simulink model "gate_V29_2016a"  (R2016a)
settings: Solver=FixedStepDiscrete  StartTime=0.0  StopTime=100  FixedStep=0.001  RelTol=1e-3
values from neighbouring files (symbols this model uses):
  CLOSE_CHAR = CHAR_STAR    [GateParameter.m]
  CLOSE_SIDE_VALUE = single(4*ONE_SEC)    [GateParameter.m]
  CLOSED = uint8(12)    [GateParameter.m]
  CLOSING = uint8(13)    [GateParameter.m]
  NO_PASS_ASSIG = uint8(6)    [GateParameter.m]
  OPEN = uint8(14)    [GateParameter.m]
  OPEN_CHAR = CHAR_HASH    [GateParameter.m]
  OPEN_SIDE_VALUE = uint16(4*ONE_SEC)    [GateParameter.m]
  OPENING = uint8(11)    [GateParameter.m]
  OPENING_ACTIVE = uint8(1)    [GateParameter.m]
  PASS_ASSIGNED = uint8(7)    [GateParameter.m]
  PASS_INCOMPLETE = uint8(8)    [GateParameter.m]
  PASS_TIMEOUT = uint16(10*ONE_SEC)    [GateParameter.m]
  PASSWORD_VERIFIED = uint8(5)    [GateParameter.m]
  TIMEOUT = uint8(9)    [GateParameter.m]
  WRONG_PASSWORD = uint8(2)    [GateParameter.m]
undefined symbols (not defined in the file or in its folder): OPEN_TIMEOUT, sigbuilder_block
libraries: simulink x9, arduinolib x5
(part 1 of 3)
settings Model: ExtModeTrigDuration=2  Creator=omaro  LastModifiedBy=oelkawok
settings DataIOCC: SaveFormat=StructureWithTime
settings OptimizationCC: DefaultParameterBehavior=Inlined  StateBitsets=on  DataBitsets=on  LifeSpan=1  MaxStackSize=64
settings DebuggingCC: ConsistencyChecking=error  ArrayBoundsChecking=error  SignalInfNanChecking=error  SignalRangeChecking=error  ReadBeforeWriteMsg=EnableAllAsError  WriteAfterWriteMsg=EnableAllAsError  WriteAfterReadMsg=EnableAllAsError  AlgebraicLoopMsg=error  ArtificialAlgebraicLoopMsg=error  BlockPriorityViolationMsg=error  MinStepSizeMsg=error  CheckMatrixSingularityMsg=error  ParameterUnderflowMsg=error  ParameterPrecisionLossMsg=error  UnderSpecifiedDataTypeMsg=warning  UnnecessaryDatatypeConvMsg=warning  VectorMatrixConversionMsg=error  SignalLabelMismatchMsg=error  SFcnCompatibilityMsg=warning  UniqueDataStoreMsg=error  BusObjectLabelMismatch=error  RootOutportRequireBusObject=error  ModelReferenceIOMsg=error  ModelReferenceVersionMismatchMessage=error  ModelReferenceIOMismatchMessage=error  ModelReferenceDataLoggingMessage=error  StrictBusMsg=ErrorOnBusTreatedAsVector  SFUnexpectedBacktrackingDiag=error  SFInvalidInputDataAccessInChartInitDiag=error  SFNoUnconditionalDefaultTransitionDiag=error  SFTransitionOutsideNaturalParentDiag=error  SFUndirectedBroadcastEventsDiag=error  SFTransitionActionBeforeConditionDiag=error  SFOutputUsedAsStateInMooreChartDiag=warning
settings SFSimCC: SimCustomHeaderCode=|
  | //#include "C:\Users\omaro\Desktop\Gate_Model\Arduino\Arduino.h"
  | //#include "Keypad\Keypad.h"
settings RTWCC: SystemTargetFile=ert.tlc  TemplateMakefile=ert_default_tmf  GenerateReport=on  RTWUseSimCustomCode=on  Toolchain=Arduino AVR v1.6.1  BuildConfiguration=Faster Runs  LaunchReport=on
settings RTWCC: CustomHeaderCode=|
  | //#include "C:\Users\omaro\Desktop\Gate_Model\Arduino\Arduino.h"
  | //#include "Keypad\Keypad.h"
settings CodeAppCC: ForceParamTrailComments=on  OperatorAnnotations=on  MATLABSourceComments=on
settings ERTTargetCC: ERTCustomFileBanners=off  SupportContinuousTime=on  SupportNonInlinedSFcns=on  SupportVariableSizeSignals=on  ModelStepFunctionPrototypeControlCompliant=off  RootIOFormat=Structure reference  ERTCustomFileTemplate=example_file_process.tlc

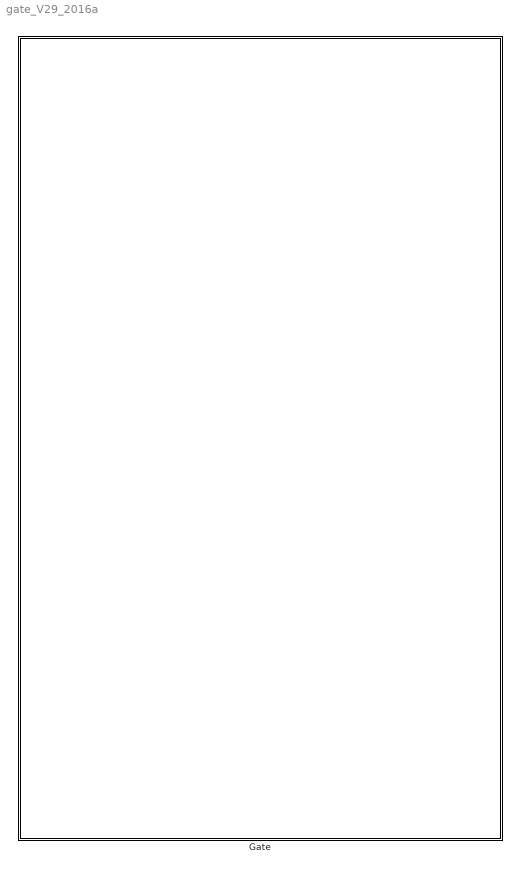
== system gate_V29_2016a ==
  Gate : Subsystem

== system gate_V29_2016a/Gate ==
  GATE : Subsystem  Variant=on  VariantControl=Variant1  OverrideUsingVariant=VariantB
  HardwareInputWrapper : Subsystem  Variant=on  VariantControl=Variant1  OverrideUsingVariant=MIL_VariantB
  outputPinsBlock : Subsystem  Variant=on  VariantControl=Variant1  OverrideUsingVariant=MIL_ALL_Variants
  wires:
  HardwareInputWrapper/9(SimuOutputLimits) -> outputPinsBlock/9(SimuOutputLimits)
  HardwareInputWrapper/2(BtCharReceived) -> GATE/2("BtCharReceived ")  "BtCharReceived_UINT8"
  HardwareInputWrapper/3(PassSwPressedOut) -> GATE/3("PassSwPressedOut ")  "PassSwPressedOut_BOOL"
  HardwareInputWrapper/4(rightCurrentRaw) -> GATE/4(rightCurrentRaw)  "rightSpeedRaw_UINT16"
  HardwareInputWrapper/6(RFPressedOut) -> GATE/6("RFPressedOut ")  "RFPressedOut_UINT8"
  GATE/1(" greenLedPin") -> outputPinsBlock/1(greenLedPin)  "greenLedPin_BOOL"
  GATE/2(" redLedPin") -> outputPinsBlock/2(redLedPin)  "redLedPin_BOOL"
  GATE/3(" yellowLedPin") -> outputPinsBlock/3(yellowLed)  "yellowLedPin_BOOL"
  GATE/4(" LeftMotorPin") -> outputPinsBlock/4(leftMotorPin)  "LeftMotorPin_BOOL"
  GATE/5(" CommonMotorPin") -> outputPinsBlock/5(commonMotorPin)  "ComMotorPin_BOOL"
  GATE/6(" rightMotorPin") -> outputPinsBlock/6(rightMotorPin)  "RightMotorPin_BOOL"
  GATE/7(" bulbPin") -> outputPinsBlock/7(bulbPin)  "bulbPin_BOOL"
  GATE/8(" BT_TxChar") -> outputPinsBlock/8(BT_TxChar)  "BT_TxChar_UINT8"
  HardwareInputWrapper/5(leftCurrentRaw) -> GATE/5(leftCurrentRaw)  "leftSpeedRaw_UINT16"
  HardwareInputWrapper/1(KpCharPressed) -> GATE/1("KpCharPressed ")  "KpCharPressed_UINT8"
  GATE/10(passwordOut) -> [passwordOut]  "passwordRAM_UINT8_6D"
  GATE/9(passAssigned) -> [passAssigned]  "passAssignedRAM_BOOL"
  [passwordOut] -> HardwareInputWrapper/2(passwordIn)
  [passAssigned] -> HardwareInputWrapper/1(passAssigned)
  HardwareInputWrapper/7(passwordOut) -> GATE/7(passwordIn)  "passwordEEPROM_UINT8_6D"
  HardwareInputWrapper/8(passAssignedOut) -> GATE/8(passAssignedIn)  "passAssignedEEPROM_BOOL"

== system gate_V29_2016a/Gate/GATE ==
  "KpCharPressed " : Inport
  "BtCharReceived " : Inport  Port=2
  "PassSwPressedOut " : Inport  Port=3
  rightCurrentRaw : Inport  Port=4
  leftCurrentRaw : Inport  Port=5
  "RFPressedOut " : Inport  Port=6
  passwordIn : Inport  Port=7
  passAssignedIn : Inport  Port=8
  GATE_A : Subsystem  VariantControl=VariantA
  GATE_B : Subsystem  VariantControl=VariantB
  GATE_C : Subsystem  VariantControl=VariantC
  " greenLedPin" : Outport
  " redLedPin" : Outport  Port=2
  " yellowLedPin" : Outport  Port=3
  " LeftMotorPin" : Outport  Port=4
  " CommonMotorPin" : Outport  Port=5
  " rightMotorPin" : Outport  Port=6
  " bulbPin" : Outport  Port=7
  " BT_TxChar" : Outport  Port=8
  passAssigned : Outport  Port=9
  passwordOut : Outport  Port=10

== system gate_V29_2016a/Gate/HardwareInputWrapper ==
  passAssigned : Inport
  passwordIn : Inport  Port=2
  HIL : Subsystem  VariantControl=HardwareInloopInputs
  MIL_Gate_A : Subsystem  VariantControl=MIL_VariantA
  MIL_Gate_B : Subsystem  VariantControl=MIL_VariantB
  MIL_Gate_C : Subsystem  VariantControl=MIL_VariantC
  KpCharPressed : Outport
  BtCharReceived : Outport  Port=2
  PassSwPressedOut : Outport  Port=3
  rightCurrentRaw : Outport  Port=4
  leftCurrentRaw : Outport  Port=5
  RFPressedOut : Outport  Port=6
  passwordOut : Outport  Port=7
  passAssignedOut : Outport  Port=8
  SimuOutputLimits : Outport  Port=9

== system gate_V29_2016a/Gate/outputPinsBlock ==
  greenLedPin : Inport
  redLedPin : Inport  Port=2
  yellowLed : Inport  Port=3
  leftMotorPin : Inport  Port=4
  commonMotorPin : Inport  Port=5
  rightMotorPin : Inport  Port=6
  bulbPin : Inport  Port=7
  BT_TxChar : Inport  Port=8
  SimuOutputLimits : Inport  Port=9
  HIL : Subsystem  VariantControl=HardwareInLoopOutputs
  MIL : Subsystem  Variant=on  VariantControl=MIL_ALL_Variants  OverrideUsingVariant=VisualOutputs

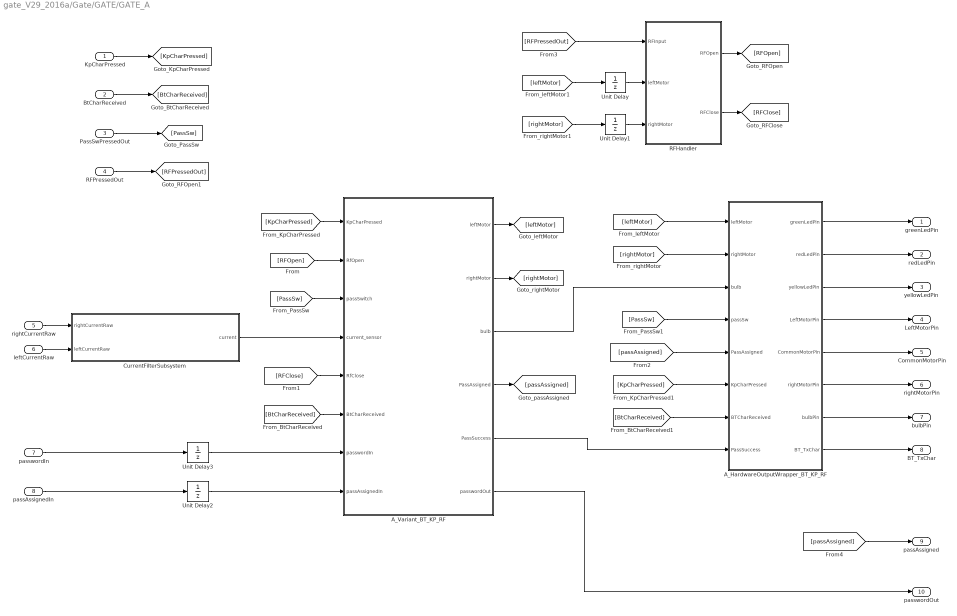
== system gate_V29_2016a/Gate/GATE/GATE_A ==
  KpCharPressed : Inport
  BtCharReceived : Inport  Port=2
  PassSwPressedOut : Inport  Port=3
  RFPressedOut : Inport  Port=4
  rightCurrentRaw : Inport  Port=5
  leftCurrentRaw : Inport  Port=6
  passwordIn : Inport  Port=7
  passAssignedIn : Inport  Port=8
  A_HardwareOutputWrapper_BT_KP_RF : Subsystem  VariantControl=Variant1
  A_Variant_BT_KP_RF : Subsystem  VariantControl=Variant
  CurrentFilterSubsystem : Subsystem
  RFHandler : Subsystem
  Unit Delay{,1..3} (4 alike) : UnitDelay  SampleTime=-1
  greenLedPin : Outport
  redLedPin : Outport  Port=2
  yellowLedPin : Outport  Port=3
  LeftMotorPin : Outport  Port=4
  CommonMotorPin : Outport  Port=5
  rightMotorPin : Outport  Port=6
  bulbPin : Outport  Port=7
  BT_TxChar : Outport  Port=8
  passAssigned : Outport  Port=9
  passwordOut : Outport  Port=10
  wires:
  RFHandler/1(RFOpen) -> [RFOpen]
  RFHandler/2(RFClose) -> [RFClose]
  [RFPressedOut] -> RFHandler/1(RFInput)
  RFPressedOut/1 -> [RFPressedOut]
  PassSwPressedOut/1 -> [PassSw]
  [leftMotor] -> Unit Delay/1
  Unit Delay/1 -> RFHandler/2(leftMotor)
  [rightMotor] -> Unit Delay1/1
  Unit Delay1/1 -> RFHandler/3(rightMotor)
  BtCharReceived/1 -> [BtCharReceived]
  KpCharPressed/1 -> [KpCharPressed]
  [RFOpen] -> A_Variant_BT_KP_RF/2(RfOpen)
  [PassSw] -> A_Variant_BT_KP_RF/3(passSwitch)
  [RFClose] -> A_Variant_BT_KP_RF/5(RfClose)
  [BtCharReceived] -> A_Variant_BT_KP_RF/6(BtCharReceived)
  A_Variant_BT_KP_RF/1(leftMotor) -> [leftMotor]
  A_Variant_BT_KP_RF/2(rightMotor) -> [rightMotor]
  [KpCharPressed] -> A_Variant_BT_KP_RF/1(KpCharPressed)
  [leftMotor] -> A_HardwareOutputWrapper_BT_KP_RF/1(leftMotor)
  [rightMotor] -> A_HardwareOutputWrapper_BT_KP_RF/2(rightMotor)
  A_Variant_BT_KP_RF/3(bulb) -> A_HardwareOutputWrapper_BT_KP_RF/3(bulb)
  [PassSw] -> A_HardwareOutputWrapper_BT_KP_RF/4(passSw)
  [KpCharPressed] -> A_HardwareOutputWrapper_BT_KP_RF/6(KpCharPressed)
  [BtCharReceived] -> A_HardwareOutputWrapper_BT_KP_RF/7(BTCharReceived)
  A_HardwareOutputWrapper_BT_KP_RF/1(greenLedPin) -> greenLedPin/1
  A_HardwareOutputWrapper_BT_KP_RF/2(redLedPin) -> redLedPin/1
  A_HardwareOutputWrapper_BT_KP_RF/3(yellowLedPin) -> yellowLedPin/1
  A_HardwareOutputWrapper_BT_KP_RF/4(LeftMotorPin) -> LeftMotorPin/1
  A_HardwareOutputWrapper_BT_KP_RF/5(CommonMotorPin) -> CommonMotorPin/1
  A_HardwareOutputWrapper_BT_KP_RF/6(rightMotorPin) -> rightMotorPin/1
  A_HardwareOutputWrapper_BT_KP_RF/7(bulbPin) -> bulbPin/1
  A_HardwareOutputWrapper_BT_KP_RF/8(BT_TxChar) -> BT_TxChar/1
  A_Variant_BT_KP_RF/4(PassAssigned) -> [passAssigned]
  [passAssigned] -> A_HardwareOutputWrapper_BT_KP_RF/5(PassAssigned)
  Unit Delay2/1 -> A_Variant_BT_KP_RF/8(passAssignedIn)
  Unit Delay3/1 -> A_Variant_BT_KP_RF/7(passwordIn)
  A_Variant_BT_KP_RF/5(PassSuccess) -> A_HardwareOutputWrapper_BT_KP_RF/8(PassSuccess)
  CurrentFilterSubsystem/1(current) -> A_Variant_BT_KP_RF/4(current_sensor)
  rightCurrentRaw/1 -> CurrentFilterSubsystem/1(rightCurrentRaw)
  leftCurrentRaw/1 -> CurrentFilterSubsystem/2(leftCurrentRaw)
  passAssignedIn/1 -> Unit Delay2/1
  passwordIn/1 -> Unit Delay3/1
  A_Variant_BT_KP_RF/6(passwordOut) -> passwordOut/1
  [passAssigned] -> passAssigned/1

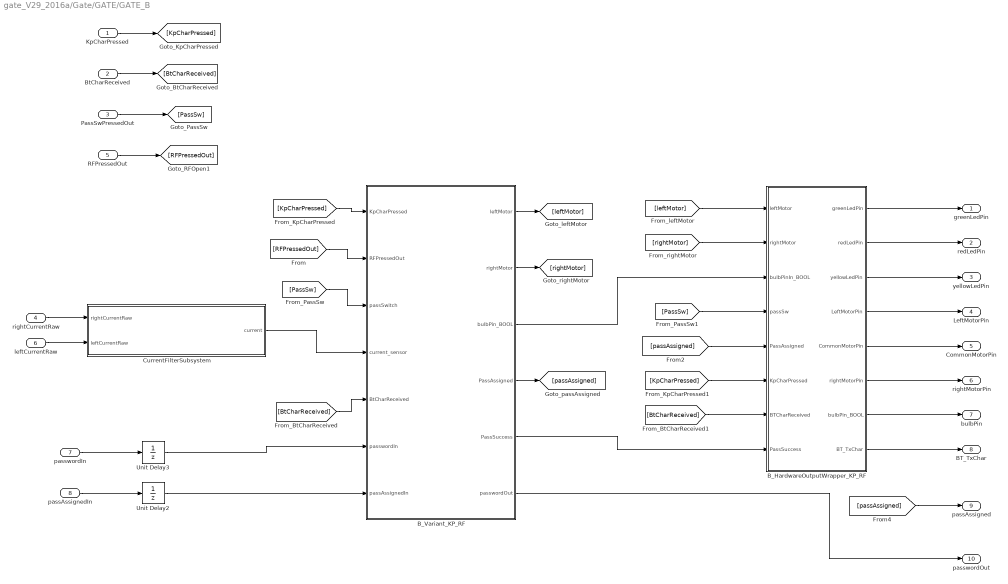
== system gate_V29_2016a/Gate/GATE/GATE_B ==
  KpCharPressed : Inport
  BtCharReceived : Inport  Port=2
  PassSwPressedOut : Inport  Port=3
  rightCurrentRaw : Inport  Port=4
  RFPressedOut : Inport  Port=5
  leftCurrentRaw : Inport  Port=6
  passwordIn : Inport  Port=7
  passAssignedIn : Inport  Port=8
  B_HardwareOutputWrapper_KP_RF : Subsystem  VariantControl=Variant2
  B_Variant_KP_RF : Subsystem  VariantControl=Variant2
  CurrentFilterSubsystem : Subsystem
  Unit Delay2 : UnitDelay  SampleTime=-1
  Unit Delay3 : UnitDelay  SampleTime=-1
  greenLedPin : Outport
  redLedPin : Outport  Port=2
  yellowLedPin : Outport  Port=3
  LeftMotorPin : Outport  Port=4
  CommonMotorPin : Outport  Port=5
  rightMotorPin : Outport  Port=6
  bulbPin : Outport  Port=7
  BT_TxChar : Outport  Port=8
  passAssigned : Outport  Port=9
  passwordOut : Outport  Port=10
  wires:
  CurrentFilterSubsystem/1(current) -> B_Variant_KP_RF/4(current_sensor)
  B_Variant_KP_RF/5(PassSuccess) -> B_HardwareOutputWrapper_KP_RF/8(PassSuccess)
  Unit Delay3/1 -> B_Variant_KP_RF/6(passwordIn)
  Unit Delay2/1 -> B_Variant_KP_RF/7(passAssignedIn)
  B_Variant_KP_RF/6(passwordOut) -> passwordOut/1
  [passAssigned] -> B_HardwareOutputWrapper_KP_RF/5(PassAssigned)
  B_Variant_KP_RF/4(PassAssigned) -> [passAssigned]
  B_HardwareOutputWrapper_KP_RF/8(BT_TxChar) -> BT_TxChar/1
  B_HardwareOutputWrapper_KP_RF/7(bulbPin_BOOL) -> bulbPin/1
  B_HardwareOutputWrapper_KP_RF/6(rightMotorPin) -> rightMotorPin/1
  B_HardwareOutputWrapper_KP_RF/5(CommonMotorPin) -> CommonMotorPin/1
  B_HardwareOutputWrapper_KP_RF/4(LeftMotorPin) -> LeftMotorPin/1
  B_HardwareOutputWrapper_KP_RF/3(yellowLedPin) -> yellowLedPin/1
  B_HardwareOutputWrapper_KP_RF/2(redLedPin) -> redLedPin/1
  B_HardwareOutputWrapper_KP_RF/1(greenLedPin) -> greenLedPin/1
  [BtCharReceived] -> B_HardwareOutputWrapper_KP_RF/7(BTCharReceived)
  [KpCharPressed] -> B_HardwareOutputWrapper_KP_RF/6(KpCharPressed)
  [PassSw] -> B_HardwareOutputWrapper_KP_RF/4(passSw)
  B_Variant_KP_RF/3(bulbPin_BOOL) -> B_HardwareOutputWrapper_KP_RF/3(bulbPinIn_BOOL)
  [rightMotor] -> B_HardwareOutputWrapper_KP_RF/2(rightMotor)
  [leftMotor] -> B_HardwareOutputWrapper_KP_RF/1(leftMotor)
  [KpCharPressed] -> B_Variant_KP_RF/1(KpCharPressed)
  B_Variant_KP_RF/2(rightMotor) -> [rightMotor]
  B_Variant_KP_RF/1(leftMotor) -> [leftMotor]
  [BtCharReceived] -> B_Variant_KP_RF/5(BtCharReceived)
  rightCurrentRaw/1 -> CurrentFilterSubsystem/1(rightCurrentRaw)
  [PassSw] -> B_Variant_KP_RF/3(passSwitch)
  [RFPressedOut] -> B_Variant_KP_RF/2(RFPressedOut)
  KpCharPressed/1 -> [KpCharPressed]
  BtCharReceived/1 -> [BtCharReceived]
  PassSwPressedOut/1 -> [PassSw]
  RFPressedOut/1 -> [RFPressedOut]
  leftCurrentRaw/1 -> CurrentFilterSubsystem/2(leftCurrentRaw)
  [passAssigned] -> passAssigned/1
  passAssignedIn/1 -> Unit Delay2/1
  passwordIn/1 -> Unit Delay3/1

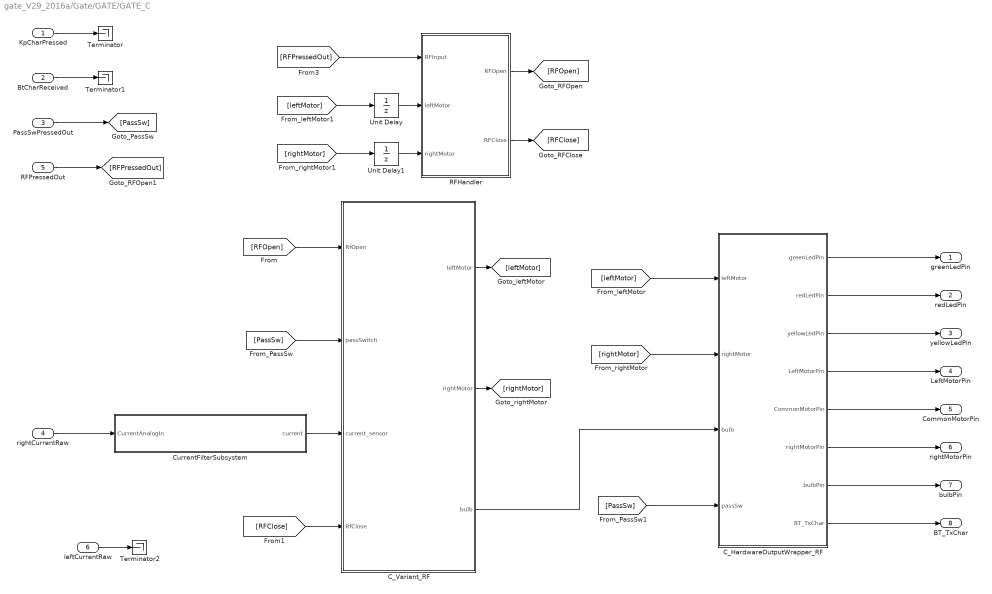
== system gate_V29_2016a/Gate/GATE/GATE_C ==
  KpCharPressed : Inport
  BtCharReceived : Inport  Port=2
  PassSwPressedOut : Inport  Port=3
  rightCurrentRaw : Inport  Port=4
  RFPressedOut : Inport  Port=5
  leftCurrentRaw : Inport  Port=6
  C_HardwareOutputWrapper_RF : Subsystem  VariantControl=Variant3
  C_Variant_RF : Subsystem  VariantControl=Variant3
  CurrentFilterSubsystem : Subsystem
  RFHandler : Subsystem
  Terminator : Terminator
  Terminator1 : Terminator
  Terminator2 : Terminator
  Unit Delay : UnitDelay  SampleTime=-1
  Unit Delay1 : UnitDelay  SampleTime=-1
  greenLedPin : Outport
  redLedPin : Outport  Port=2
  yellowLedPin : Outport  Port=3
  LeftMotorPin : Outport  Port=4
  CommonMotorPin : Outport  Port=5
  rightMotorPin : Outport  Port=6
  bulbPin : Outport  Port=7
  BT_TxChar : Outport  Port=8
  wires:
  RFHandler/1(RFOpen) -> [RFOpen]
  RFHandler/2(RFClose) -> [RFClose]
  [RFPressedOut] -> RFHandler/1(RFInput)
  RFPressedOut/1 -> [RFPressedOut]
  PassSwPressedOut/1 -> [PassSw]
  [leftMotor] -> Unit Delay/1
  Unit Delay/1 -> RFHandler/2(leftMotor)
  [rightMotor] -> Unit Delay1/1
  Unit Delay1/1 -> RFHandler/3(rightMotor)
  BtCharReceived/1 -> Terminator1/1
  KpCharPressed/1 -> Terminator/1
  [RFOpen] -> C_Variant_RF/1(RfOpen)
  [PassSw] -> C_Variant_RF/2(passSwitch)
  rightCurrentRaw/1 -> CurrentFilterSubsystem/1(CurrentAnalogIn)
  [RFClose] -> C_Variant_RF/4(RfClose)
  C_Variant_RF/1(leftMotor) -> [leftMotor]
  C_Variant_RF/2(rightMotor) -> [rightMotor]
  [leftMotor] -> C_HardwareOutputWrapper_RF/1(leftMotor)
  [rightMotor] -> C_HardwareOutputWrapper_RF/2(rightMotor)
  C_Variant_RF/3(bulb) -> C_HardwareOutputWrapper_RF/3(bulb)
  [PassSw] -> C_HardwareOutputWrapper_RF/4(passSw)
  C_HardwareOutputWrapper_RF/1(greenLedPin) -> greenLedPin/1
  C_HardwareOutputWrapper_RF/2(redLedPin) -> redLedPin/1
  C_HardwareOutputWrapper_RF/3(yellowLedPin) -> yellowLedPin/1
  C_HardwareOutputWrapper_RF/4(LeftMotorPin) -> LeftMotorPin/1
  C_HardwareOutputWrapper_RF/5(CommonMotorPin) -> CommonMotorPin/1
  C_HardwareOutputWrapper_RF/6(rightMotorPin) -> rightMotorPin/1
  C_HardwareOutputWrapper_RF/7(bulbPin) -> bulbPin/1
  C_HardwareOutputWrapper_RF/8(BT_TxChar) -> BT_TxChar/1
  CurrentFilterSubsystem/1(current) -> C_Variant_RF/3(current_sensor)
  leftCurrentRaw/1 -> Terminator2/1

== system gate_V29_2016a/Gate/HardwareInputWrapper/HIL ==
  passAssigned : Inport
  passwordIn : Inport  Port=2
  Analog Input : arduinolib/Analog Input  pinNumber=6  sampleTime=-1  blockPlatform=arduino
  Analog Input1 : arduinolib/Analog Input  pinNumber=7  sampleTime=-1  blockPlatform=arduino
  BluetoothWrapper : Subsystem  Commented=on
  ButtonsPressed : Subsystem
  EEPROM : Subsystem
  KeypadWrapper : Subsystem
  S-Function Builder1 : S-Function  Commented=on  InitFcn=try, set_param(gcb,'FunctionName','CurrentA_Lib'), end  FunctionName=CurrentA_Lib  SFunctionModules=CurrentA_Lib_wrapper  SFunctionDeploymentMode=off  EnableBusSupport=off  mask(S-Function Builder){}
      mask help: Simulink:masks:SFunctionBuilder_MD
      mask init:
      | val = [[.9  0.75 0.75 1 1 .9 .9 1]',[1 1 0.75 0.75 .9 .9 1 .9]'];try , sys = get_param(gcb,'SfunBuilderFcnName');if isempty(sys), sys = get_param(gcb,'FunctionName'); end,catch, sys = get_param(gcb,'FunctionName'); end
      settings: CopyFcn=set_param(gcbh,'LinkStatus','none')  PreSaveFcn=try, set_param(gcb,'FunctionName','CurrentA_Lib'), end  OpenFcn=sfunctionwizard(gcbh)
      mask Display: plot(val(:,1),val(:,2)),disp(sys), port_label('output',1,'currentValue')
  S-Function Builder2 : S-Function  Commented=on  InitFcn=try, set_param(gcb,'FunctionName','printingBlock'), end  FunctionName=printingBlock  SFunctionModules=printingBlock_wrapper  SFunctionDeploymentMode=off  EnableBusSupport=off  mask(S-Function Builder){}
      mask help: Simulink:masks:SFunctionBuilder_MD
      mask init: same as gate_V29_2016a/Gate/HardwareInputWrapper/HIL/S-Function Builder1
      settings: CopyFcn=set_param(gcbh,'LinkStatus','none')  PreSaveFcn=try, set_param(gcb,'FunctionName','printingBlock'), end  OpenFcn=sfunctionwizard(gcbh)
      mask Display: plot(val(:,1),val(:,2)),disp(sys), port_label('input',1,'uint8ToPrint')
  currentSensorWrapperOld : Subsystem  Commented=on
  KpCharPressed : Outport
  BtCharReceived : Outport  Port=2
  PassSwPressedOut : Outport  Port=3
  rightCurrentRaw : Outport  Port=4
  leftCurrentRaw : Outport  Port=5
  RFPressedOut : Outport  Port=6
  passwordOut : Outport  Port=7
  passAssignedOut : Outport  Port=8
  wires:
  BluetoothWrapper/1(bluetoothCharPressed) -> BtCharReceived/1
  Analog Input/1 -> rightCurrentRaw/1  "rightMotorSpeed"
  KeypadWrapper/1(charPressed) -> S-Function Builder2/1, KpCharPressed/1
  ButtonsPressed/2(SWPressed) -> [PassSwPressedOut], PassSwPressedOut/1
  ButtonsPressed/1(RFPressed) -> RFPressedOut/1
  Analog Input1/1 -> leftCurrentRaw/1  "leftMotorSpeed"
  [PassSwPressedOut] -> EEPROM/1(passSw)
  passAssigned/1 -> EEPROM/2(passAssigned)
  passwordIn/1 -> EEPROM/3(passwordIn)
  EEPROM/1(passwordOut) -> passwordOut/1
  EEPROM/2(passAssignedOut) -> passAssignedOut/1

== system gate_V29_2016a/Gate/HardwareInputWrapper/MIL_Gate_A ==
  Bus Creator : BusCreator  Inputs=16
  Mux : Mux  Inputs=6
  Quantizer{,1..3} (4 alike) : Quantizer  QuantizationInterval=-1
  Signal Builder : Subsystem  Tag=STV Subsys  LoadFcn=sigbuilder_block('load');  StartFcn=sigbuilder_block('start');  StopFcn=sigbuilder_block('stop');  ClipboardFcn=sigbuilder_block('clipboard');  mask(Sigbuilder block){}
      mask help: Simulink:masks:SigbuilderBlock_MD
      mask init:
      | if ~strcmp(get_param(bdroot(gcbh),'SimulationStatus'),'stopped') tuvar = sigbuilder_block('maskInit'); end
      settings: CopyFcn=sigbuilder_block('copy');  DeleteFcn=sigbuilder_block('delete');  ModelCloseFcn=sigbuilder_block('modelClose');  PreSaveFcn=sigbuilder_block('preSave');  PauseFcn=sigbuilder_block('pause');  ContinueFcn=sigbuilder_block('continue');  NameChangeFcn=sigbuilder_block('namechange');  OpenFcn=sigbuilder_block('open',[6 28.2 1012.8 484.8 ]);  CloseFcn=sigbuilder_block('close');
      mask Display: plot(0, 0, 100, 100,[2, 2, 32, 32, 2], [68, 8, 8, 68, 68],[32, 2], [38, 38], [32, 19, 2],[53, 60, 44], [32, 17, 17, 2],[16, 16, 31, 31]);txt = getActiveGroup(gcbh);text(2, 100, txt,'verticalAlignment', 'top');
  KpCharPressed : Outport
  BtCharReceived : Outport  Port=2
  PassSwPressedOut : Outport  Port=3
  RFPressedOut : Outport  Port=4
  rightCurrentRaw : Outport  Port=5
  leftCurrentRaw : Outport  Port=6
  SimuOutputLimits : Outport  Port=7
  passwordOut : Outport  Port=8
  passAssignedOut : Outport  Port=9
  wires:
  Signal Builder/2(BT_input) -> Quantizer1/1
  Signal Builder/4(SW_input) -> Quantizer3/1
  Signal Builder/1(Kp_input) -> Quantizer/1
  Signal Builder/3(RF_input) -> Quantizer2/1
  Quantizer/1 -> KpCharPressed/1
  Quantizer1/1 -> BtCharReceived/1
  Quantizer2/1 -> RFPressedOut/1
  Quantizer3/1 -> PassSwPressedOut/1
  Bus Creator/1 -> SimuOutputLimits/1
  Signal Builder/17(GreenLedMax) -> Bus Creator/11  "GreenLedMax"
  Signal Builder/16(YellowLedMin) -> Bus Creator/10  "YellowLedMin"
  Signal Builder/18(GreenLedMin) -> Bus Creator/12  "GreenLedMin"
  Signal Builder/15(YellowLedMax) -> Bus Creator/9  "YellowLedMax"
  Signal Builder/19(BulbMax) -> Bus Creator/13  "BulbMax"
  Signal Builder/14(RedLedMin) -> Bus Creator/8  "RedLedMin"
  Signal Builder/20(BulbMin) -> Bus Creator/14  "BulbMin"
  Signal Builder/21(BT_ouputMax) -> Bus Creator/15  "BT_outputMax"
  Signal Builder/13(RedLedMax) -> Bus Creator/7  "RedLedMax"
  Signal Builder/12(MCMin) -> Bus Creator/6  "MCMin"
  Signal Builder/11(MCMax) -> Bus Creator/5  "MCMax"
  Signal Builder/10(MRMin) -> Bus Creator/4  "MRMin"
  Signal Builder/9(MRMax) -> Bus Creator/3  "MRMax"
  Signal Builder/8(MLMin) -> Bus Creator/2  "MLMin"
  Signal Builder/7(MLMax) -> Bus Creator/1  "MLMax"
  Signal Builder/22(BT_outputMin) -> Bus Creator/16  "BT_outputMin"
  Signal Builder/5(rightSpeedRaw) -> rightCurrentRaw/1
  Signal Builder/6(leftSpeedRaw) -> leftCurrentRaw/1
  Signal Builder/23(PassAssignedEEPROM) -> passAssignedOut/1
  Mux/1 -> passwordOut/1
  Signal Builder/24(Pass1) -> Mux/1
  Signal Builder/25(Pass2) -> Mux/2
  Signal Builder/26(Pass3) -> Mux/3
  Signal Builder/27(Pass4) -> Mux/4
  Signal Builder/28(Pass5) -> Mux/5
  Signal Builder/29(Pass6) -> Mux/6

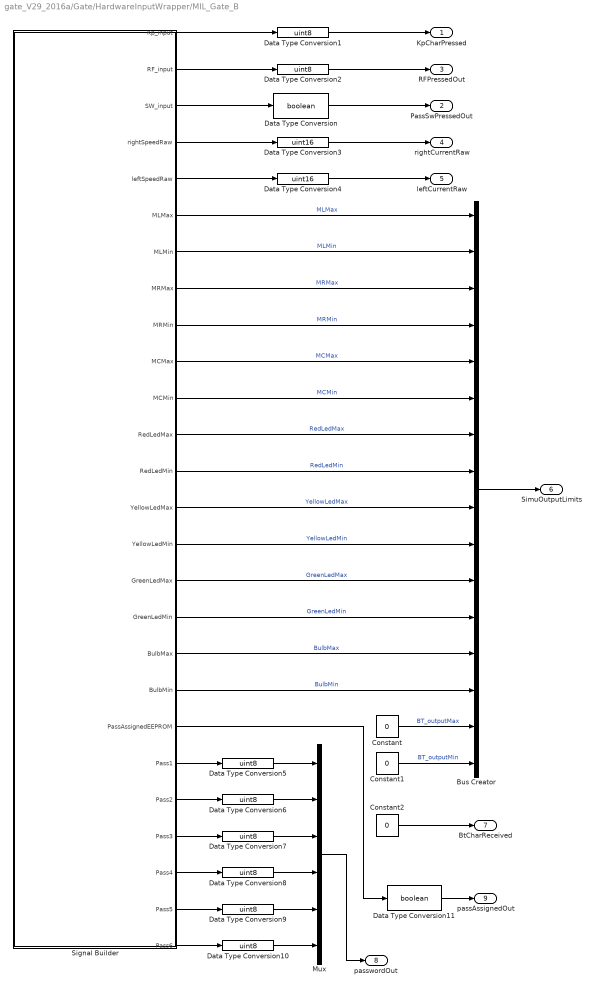
== system gate_V29_2016a/Gate/HardwareInputWrapper/MIL_Gate_B ==
  Bus Creator : BusCreator  Inputs=16
  Constant : Constant  Value=0
  Constant1 : Constant  Value=0
  Constant2 : Constant  Value=0
  Data Type Conversion : DataTypeConversion  OutDataTypeStr=boolean
  Data Type Conversion{1..2,5..10} (8 alike) : DataTypeConversion  OutDataTypeStr=uint8
  Data Type Conversion11 : DataTypeConversion  OutDataTypeStr=boolean
  Data Type Conversion3 : DataTypeConversion  OutDataTypeStr=uint16
  Data Type Conversion4 : DataTypeConversion  OutDataTypeStr=uint16
  Mux : Mux  Inputs=6
  Signal Builder : Subsystem  Tag=STV Subsys  LoadFcn=sigbuilder_block('load',[-1152 0 1152 592.8 ]);  StartFcn=sigbuilder_block('start');  StopFcn=sigbuilder_block('stop');  ClipboardFcn=sigbuilder_block('clipboard');  mask(Sigbuilder block){}
      mask help: Simulink:masks:SigbuilderBlock_MD
      mask init:
      | if ~strcmp(get_param(bdroot(gcbh),'SimulationStatus'),'stopped') tuvar = sigbuilder_block('maskInit'); end
      settings: CopyFcn=sigbuilder_block('copy');  DeleteFcn=sigbuilder_block('delete');  ModelCloseFcn=sigbuilder_block('modelClose');  PreSaveFcn=sigbuilder_block('preSave');  PauseFcn=sigbuilder_block('pause');  ContinueFcn=sigbuilder_block('continue');  NameChangeFcn=sigbuilder_block('namechange');  OpenFcn=sigbuilder_block('open',[19.2 31.8 1141.2 525 ]);  CloseFcn=sigbuilder_block('close');
      mask Display: plot(0, 0, 100, 100,[2, 2, 32, 32, 2], [68, 8, 8, 68, 68],[32, 2], [38, 38], [32, 19, 2],[53, 60, 44], [32, 17, 17, 2],[16, 16, 31, 31]);txt = getActiveGroup(gcbh);text(2, 100, txt,'verticalAlignment', 'top');
  KpCharPressed : Outport
  PassSwPressedOut : Outport  Port=2